simulink model "profproject_simulation_cl"  (R2023b)
settings: Solver=FixedStepAuto  StartTime=0.0  StopTime=45  FixedStep=0.05  RelTol=1e-3
model workspace (MAT data in the model file):
  Logfile_Data_Object : robotics.ros.BagSelection (value not decoded)
  Logfile_Path = '/Users/sprinkle/work/teaching/cs3891-2023F/src/docker/mytest.bag'
  Logfile_Playback_Duration = 200
  Logfile_Playback_Offset = 100
libraries: simulink x1
settings Model: Creator=sprinkle  LastModifiedBy=marystirlingbrown
settings SolverCC: AlgebraicLoopSolver=TrustRegion
settings OptimizationCC: EnhancedBackFolding=on  PassReuseOutputArgsAs=Individual arguments  ZeroExternalMemoryAtStartup=off  ZeroInternalMemoryAtStartup=off  MaxStackSize=64  OptimizeBlockOrder=speed  DifferentSizesBufferReuse=on  OptimizationCustomize=off  EfficientTunableParamExpr=on
settings DebuggingCC: ParameterTunabilityLossMsg=error  UnconnectedInputMsg=warning  UnconnectedOutputMsg=warning  UnconnectedLineMsg=warning
settings HardwareCC: ProdBitPerPointer=32  ProdBitPerSizeT=32  ProdBitPerPtrDiffT=32  ProdLargestAtomicFloat=None  ProdIntDivRoundTo=Undefined  ProdEndianess=Unspecified  ProdWordSize=32  ProdHWDeviceType=Generic->Unspecified (assume 32-bit Generic)
settings ModelReferenceCC: EnableRefExpFcnMdlSchedulingChecks=on
settings SFSimCC: MATLABDynamicMemAlloc=off
settings RTWCC: Description=Embedded Coder  SystemTargetFile=ert.tlc  HardwareBoard=Robot Operating System (ROS)  TLCOptions="-aInlineSetEventsForThisBaseRateFcn=TLC_FALSE -aSuppressMultiTaskScheduler=TLC_FALSE  -aRateBasedStepFcn=0"  TemplateMakefile=ert_default_tmf  Toolchain=Catkin  TargetLang=C++
settings CodeAppCC: ForceParamTrailComments=on  IgnoreCustomStorageClasses=off  OperatorAnnotations=on  SimulinkDataObjDesc=on  SFDataObjDesc=on  InsertBlockDesc=on
settings ERTTargetCC: SupportContinuousTime=on

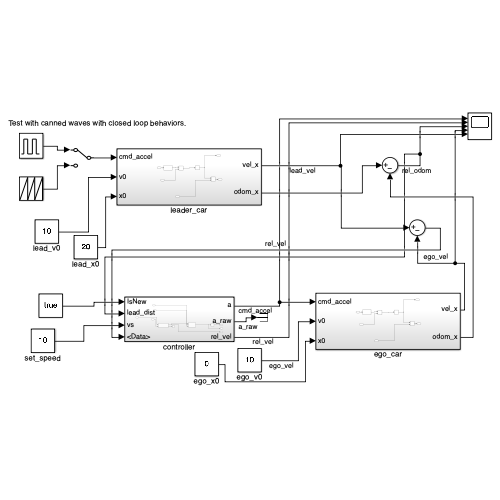
[thumbnail: Simulink's stored preview of the top-level diagram]
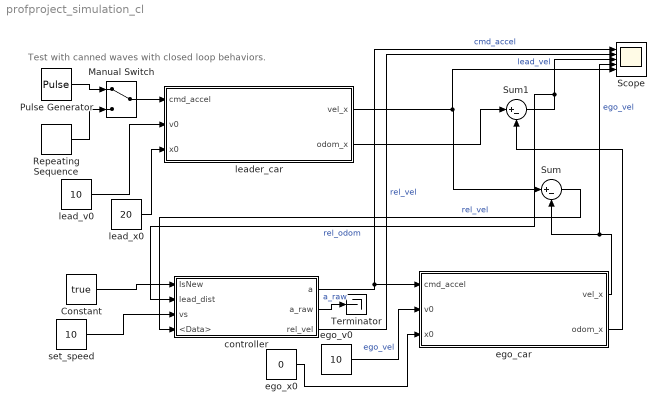
== system profproject_simulation_cl ==
  note: Test with canned waves with closed loop behaviors.
  Constant : Constant  Value=true
  Manual Switch : ManualSwitch
  Pulse Generator : DiscretePulseGenerator  PulseType=Time based  Amplitude=2  Period=10  PulseWidth=30
  Repeating Sequence : simulink/Sources/Repeating Sequence  rep_seq_t=[0 20]  rep_seq_y=[-2 2]
  Scope : Scope  NumInputPorts=5
  Sum : Sum  Inputs=|+-
  Sum1 : Sum  Inputs=|+-
  Terminator : Terminator
  controller : Subsystem
  ego_car : Subsystem
  ego_v0 : Constant  Value=10
  ego_x0 : Constant  Value=0
  lead_v0 : Constant  Value=10
  lead_x0 : Constant  Value=20
  leader_car : Subsystem
  set_speed : Constant  Value=10
  wires:
  controller/3(rel_vel) -> Scope/2  "rel_vel"
  Constant/1 -> controller/1(IsNew)
  Manual Switch/1 -> leader_car/1(cmd_accel)
  Pulse Generator/1 -> Manual Switch/1
  Repeating Sequence/1 -> Manual Switch/2
  leader_car/1(vel_x) -> Scope/5, Sum/1  "lead_vel"
  ego_car/1(vel_x) -> Sum/2, Scope/4  "ego_vel"
  Sum/1 -> controller/4(<Data>)  "rel_vel"
  lead_v0/1 -> leader_car/2(v0)
  lead_x0/1 -> leader_car/3(x0)
  ego_v0/1 -> ego_car/2(v0)  "ego_vel"
  ego_x0/1 -> ego_car/3(x0)
  controller/1(a) -> Scope/1, ego_car/1(cmd_accel)  "cmd_accel"
  leader_car/2(odom_x) -> Sum1/1
  ego_car/2(odom_x) -> Sum1/2
  Sum1/1 -> controller/2(lead_dist), Scope/3  "rel_odom"
  controller/2(a_raw) -> Terminator/1  "a_raw"
  set_speed/1 -> controller/3(vs)

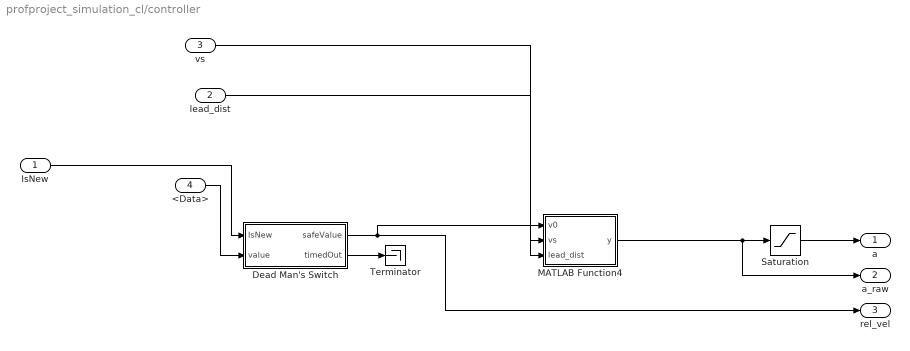
== system profproject_simulation_cl/controller ==
  IsNew : Inport
  lead_dist : Inport  Port=2
  vs : Inport  Port=3
  <Data> : Inport  Port=4
  Dead Man's Switch : Subsystem  TreatAsAtomicUnit=on  mask{stepSize=0.05, timeout=0.4}
      mask prompts: stepSize "Simulation step size"; timeout "Timeout (in seconds)"
      mask help: Interprets whether a new message has been recently received, and if so permits the data in 'value' to be passed through. Otherwise, 0 is outputted from 'safeValue'
      mask Help: Interprets whether a new message has been recently received, and if so permits the data in 'value' to be passed through. Otherwise, 0 is outputted from 'safeValue'
  MATLAB Function4 : MATLAB Function  TreatAsAtomicUnit=on
  Saturation : Saturate  UpperLimit=1.5  LowerLimit=-3
  Terminator : Terminator
  a : Outport
  a_raw : Outport  Port=2
  rel_vel : Outport  Port=3
  wires:
  IsNew/1 -> Dead Man's Switch/1(IsNew)
  Dead Man's Switch/2(timedOut) -> Terminator/1
  <Data>/1 -> Dead Man's Switch/2(value)
  Saturation/1 -> a/1
  Dead Man's Switch/1(safeValue) -> MATLAB Function4/1(v0), rel_vel/1
  vs/1 -> MATLAB Function4/2(vs)
  MATLAB Function4/1(y) -> a_raw/1, Saturation/1
  lead_dist/1 -> MATLAB Function4/3(lead_dist)

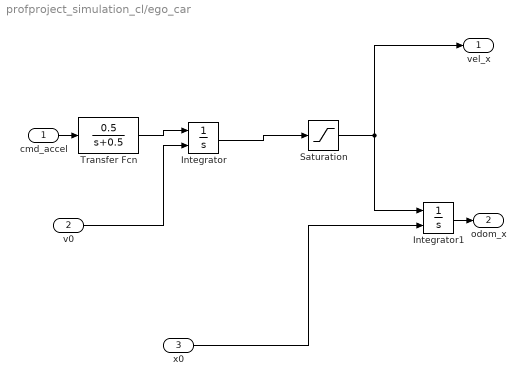
== system profproject_simulation_cl/ego_car ==
  cmd_accel : Inport
  v0 : Inport  Port=2
  x0 : Inport  Port=3
  Integrator : Integrator  InitialConditionSource=external
  Integrator1 : Integrator  InitialConditionSource=external
  Saturation : Saturate  UpperLimit=80  LowerLimit=0
  Transfer Fcn : TransferFcn  Numerator=[0.5]  Denominator=[1 0.5]
  vel_x : Outport
  odom_x : Outport  Port=2
  wires:
  v0/1 -> Integrator/2
  cmd_accel/1 -> Transfer Fcn/1
  Transfer Fcn/1 -> Integrator/1
  Integrator1/1 -> odom_x/1
  Integrator/1 -> Saturation/1
  x0/1 -> Integrator1/2
  Saturation/1 -> vel_x/1, Integrator1/1

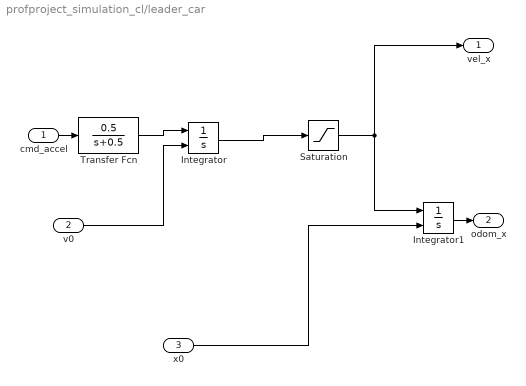
== system profproject_simulation_cl/leader_car ==
  cmd_accel : Inport
  v0 : Inport  Port=2
  x0 : Inport  Port=3
  Integrator : Integrator  InitialConditionSource=external
  Integrator1 : Integrator  InitialConditionSource=external
  Saturation : Saturate  UpperLimit=80  LowerLimit=0
  Transfer Fcn : TransferFcn  Numerator=[0.5]  Denominator=[1 0.5]
  vel_x : Outport
  odom_x : Outport  Port=2
  wires:
  v0/1 -> Integrator/2
  cmd_accel/1 -> Transfer Fcn/1
  Transfer Fcn/1 -> Integrator/1
  Integrator1/1 -> odom_x/1
  Integrator/1 -> Saturation/1
  x0/1 -> Integrator1/2
  Saturation/1 -> vel_x/1, Integrator1/1

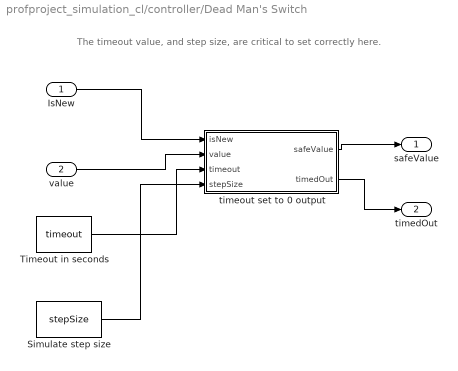
== system profproject_simulation_cl/controller/Dead Man's Switch ==
  note: The timeout value, and step size, are critical to set correctly here.
  IsNew : Inport
  value : Inport  Port=2
  Simulate step size : Constant  Value=stepSize
  Timeout in seconds : Constant  Value=timeout
  timeout set to 0 output : MATLAB Function  TreatAsAtomicUnit=on
  safeValue : Outport  InitialOutput=[0]
  timedOut : Outport  Port=2  InitialOutput=[0]
  wires:
  IsNew/1 -> timeout set to 0 output/1(isNew)
  timeout set to 0 output/1(safeValue) -> safeValue/1
  value/1 -> timeout set to 0 output/2(value)
  Timeout in seconds/1 -> timeout set to 0 output/3(timeout)
  timeout set to 0 output/2(timedOut) -> timedOut/1
  Simulate step size/1 -> timeout set to 0 output/4(stepSize)

== matlab function profproject_simulation_cl/controller/Dead Man's Switch/timeout set to 0 output ==
  settings: updateMethod=DISCRETE
  data: isNew input; safeValue output; value input; timeout input; timedOut output; stepSize input
function [safeValue,timedOut] = fcn(isNew,value,timeout,stepSize)
%#codegen
persistent sinceLastMsg
timedOut=true;
if isempty(sinceLastMsg)
    sinceLastMsg=(timeout/stepSize)+1;
end

safeValue=0;
% if no new message
% after timeout, we output 0
if( isNew == true )
    sinceLastMsg = 0;
else
    sinceLastMsg = sinceLastMsg+1;
end

% Note: we require step size as an input here, and depend on our
% system being executed at a regular rate, or bad things will happen
if( sinceLastMsg < timeout/stepSize )
    timedOut = false;
end
    
if( timedOut == false )
    safeValue = value;
end

== matlab function profproject_simulation_cl/controller/MATLAB Function4 ==
  data: v0 input; y output; vs input; lead_dist input
function y = fcn(v0, vs, lead_dist)
    v0
    vs
    lead_dist
    y = 0; % Default value if vs is equal to v0

    if vs ~= v0
        y = accel(v0, vs, lead_dist);
    end
end

function acceleration = accel(V_0, V_S, lead_dist)
    % Initialize V_C (current velocity) - you didn't provide its value in the code
    V_C = 0; % Replace 0 with the actual current velocity

    % Calculate time gap
    T_g = lead_dist / V_0;

    if T_g < 2
        acceleration = 0;
        return;
    end

    if V_S > V_C
        % Check safety and apply acceleration
        if ~safe(T_g)
            acceleration = 0;
        end
        % Calculate acceleration based on an arbitrary constant
        acceleration = (V_S - V_C) / 10;
        
        
    elseif V_S < V_C
        
        % Calculate deceleration based on an arbitrary constant
        acceleration = (V_S - V_C) / 10;
    else
        % If V_S is equal to V_C, no acceleration or deceleration
        acceleration = 0;
    end

end

function result = safe(Tau)
    % Check if the time gap is less than 2 seconds
    if Tau < 2
        result = false;
    else
        result = true;
    end
end
